simulink model "mbf_3beams"  (R2013a) (continued)
== system mbf_3beams == (continued)
      mask Display:
      | port_label('input', 1, 'd');
      | port_label('output', 1, 'q');
      | disp(['Z^-', num2str(latency)]);
  pipeline32 : Subsystem  AncestorBlock=casper_library_delays/pipeline  mask(pipeline){latency=1}
      mask help:
      | An explicitly laid-out delay line to avoid mapping to SRL16s
      | for use in pipelining to help achieve timing closure.
      mask init:
      | pipeline_init(gcb,'latency',latency);
      settings: AttributesFormatString=Latency = %<latency>
      mask Display:
      | port_label('input', 1, 'd');
      | port_label('output', 1, 'q');
      | disp(['Z^-', num2str(latency)]);
  pipeline33 : Subsystem  AncestorBlock=casper_library_delays/pipeline  mask(pipeline){latency=1}
      mask help:
      | An explicitly laid-out delay line to avoid mapping to SRL16s
      | for use in pipelining to help achieve timing closure.
      mask init:
      | pipeline_init(gcb,'latency',latency);
      settings: AttributesFormatString=Latency = %<latency>
      mask Display:
      | port_label('input', 1, 'd');
      | port_label('output', 1, 'q');
      | disp(['Z^-', num2str(latency)]);
  pipeline34 : Subsystem  AncestorBlock=casper_library_delays/pipeline  mask(pipeline){latency=2}
      mask help:
      | An explicitly laid-out delay line to avoid mapping to SRL16s
      | for use in pipelining to help achieve timing closure.
      mask init:
      | pipeline_init(gcb,'latency',latency);
      settings: AttributesFormatString=Latency = %<latency>
      mask Display:
      | port_label('input', 1, 'd');
      | port_label('output', 1, 'q');
      | disp(['Z^-', num2str(latency)]);
  pipeline35 : casper_library_delays/pipeline  latency=2
      settings: AttributesFormatString=Latency = %<latency>
  pipeline36 : Subsystem  AncestorBlock=casper_library_delays/pipeline  mask(pipeline){latency=2}
      mask help:
      | An explicitly laid-out delay line to avoid mapping to SRL16s
      | for use in pipelining to help achieve timing closure.
      mask init:
      | pipeline_init(gcb,'latency',latency);
      settings: AttributesFormatString=Latency = %<latency>
      mask Display:
      | port_label('input', 1, 'd');
      | port_label('output', 1, 'q');
      | disp(['Z^-', num2str(latency)]);
  pipeline37 : Subsystem  AncestorBlock=casper_library_delays/pipeline  mask(pipeline){latency=2}
      mask help:
      | An explicitly laid-out delay line to avoid mapping to SRL16s
      | for use in pipelining to help achieve timing closure.
      mask init:
      | pipeline_init(gcb,'latency',latency);
      settings: AttributesFormatString=Latency = %<latency>
      mask Display:
      | port_label('input', 1, 'd');
      | port_label('output', 1, 'q');
      | disp(['Z^-', num2str(latency)]);
  pipeline38 : Subsystem  AncestorBlock=casper_library_delays/pipeline  mask(pipeline){latency=2}
      mask help:
      | An explicitly laid-out delay line to avoid mapping to SRL16s
      | for use in pipelining to help achieve timing closure.
      mask init:
      | pipeline_init(gcb,'latency',latency);
      settings: AttributesFormatString=Latency = %<latency>
      mask Display:
      | port_label('input', 1, 'd');
      | port_label('output', 1, 'q');
      | disp(['Z^-', num2str(latency)]);
  pipeline39 : Subsystem  AncestorBlock=casper_library_delays/pipeline  mask(pipeline){latency=1}
      mask help:
      | An explicitly laid-out delay line to avoid mapping to SRL16s
      | for use in pipelining to help achieve timing closure.
      mask init:
      | pipeline_init(gcb,'latency',latency);
      settings: AttributesFormatString=Latency = %<latency>
      mask Display:
      | port_label('input', 1, 'd');
      | port_label('output', 1, 'q');
      | disp(['Z^-', num2str(latency)]);
  pipeline4 : Subsystem  AncestorBlock=casper_library_delays/pipeline  mask(pipeline){latency=1}
      mask help:
      | An explicitly laid-out delay line to avoid mapping to SRL16s
      | for use in pipelining to help achieve timing closure.
      mask init:
      | pipeline_init(gcb,'latency',latency);
      settings: AttributesFormatString=Latency = %<latency>
      mask Display:
      | port_label('input', 1, 'd');
      | port_label('output', 1, 'q');
      | disp(['Z^-', num2str(latency)]);
  pipeline40 : Subsystem  AncestorBlock=casper_library_delays/pipeline  mask(pipeline){latency=1}
      mask help:
      | An explicitly laid-out delay line to avoid mapping to SRL16s
      | for use in pipelining to help achieve timing closure.
      mask init:
      | pipeline_init(gcb,'latency',latency);
      settings: AttributesFormatString=Latency = %<latency>
      mask Display:
      | port_label('input', 1, 'd');
      | port_label('output', 1, 'q');
      | disp(['Z^-', num2str(latency)]);
  pipeline41 : Subsystem  AncestorBlock=casper_library_delays/pipeline  mask(pipeline){latency=1}
      mask help:
      | An explicitly laid-out delay line to avoid mapping to SRL16s
      | for use in pipelining to help achieve timing closure.
      mask init:
      | pipeline_init(gcb,'latency',latency);
      settings: AttributesFormatString=Latency = %<latency>
      mask Display:
      | port_label('input', 1, 'd');
      | port_label('output', 1, 'q');
      | disp(['Z^-', num2str(latency)]);
  pipeline42 : Subsystem  AncestorBlock=casper_library_delays/pipeline  mask(pipeline){latency=1}
      mask help:
      | An explicitly laid-out delay line to avoid mapping to SRL16s
      | for use in pipelining to help achieve timing closure.
      mask init:
      | pipeline_init(gcb,'latency',latency);
      settings: AttributesFormatString=Latency = %<latency>
      mask Display:
      | port_label('input', 1, 'd');
      | port_label('output', 1, 'q');
      | disp(['Z^-', num2str(latency)]);
  pipeline43 : Subsystem  AncestorBlock=casper_library_delays/pipeline  mask(pipeline){latency=1}
      mask help:
      | An explicitly laid-out delay line to avoid mapping to SRL16s
      | for use in pipelining to help achieve timing closure.
      mask init:
      | pipeline_init(gcb,'latency',latency);
      settings: AttributesFormatString=Latency = %<latency>
      mask Display:
      | port_label('input', 1, 'd');
      | port_label('output', 1, 'q');
      | disp(['Z^-', num2str(latency)]);
  pipeline44 : Subsystem  AncestorBlock=casper_library_delays/pipeline  mask(pipeline){latency=1}
      mask help:
      | An explicitly laid-out delay line to avoid mapping to SRL16s
      | for use in pipelining to help achieve timing closure.
      mask init:
      | pipeline_init(gcb,'latency',latency);
      settings: AttributesFormatString=Latency = %<latency>
      mask Display:
      | port_label('input', 1, 'd');
      | port_label('output', 1, 'q');
      | disp(['Z^-', num2str(latency)]);
  pipeline45 : Subsystem  AncestorBlock=casper_library_delays/pipeline  mask(pipeline){latency=1}
      mask help:
      | An explicitly laid-out delay line to avoid mapping to SRL16s
      | for use in pipelining to help achieve timing closure.
      mask init:
      | pipeline_init(gcb,'latency',latency);
      settings: AttributesFormatString=Latency = %<latency>
      mask Display:
      | port_label('input', 1, 'd');
      | port_label('output', 1, 'q');
      | disp(['Z^-', num2str(latency)]);
  pipeline46 : Subsystem  AncestorBlock=casper_library_delays/pipeline  mask(pipeline){latency=1}
      mask help:
      | An explicitly laid-out delay line to avoid mapping to SRL16s
      | for use in pipelining to help achieve timing closure.
      mask init:
      | pipeline_init(gcb,'latency',latency);
      settings: AttributesFormatString=Latency = %<latency>
      mask Display:
      | port_label('input', 1, 'd');
      | port_label('output', 1, 'q');
      | disp(['Z^-', num2str(latency)]);
  pipeline47 : Subsystem  AncestorBlock=casper_library_delays/pipeline  mask(pipeline){latency=1}
      mask help:
      | An explicitly laid-out delay line to avoid mapping to SRL16s
      | for use in pipelining to help achieve timing closure.
      mask init:
      | pipeline_init(gcb,'latency',latency);
      settings: AttributesFormatString=Latency = %<latency>
      mask Display:
      | port_label('input', 1, 'd');
      | port_label('output', 1, 'q');
      | disp(['Z^-', num2str(latency)]);
  pipeline48 : Subsystem  AncestorBlock=casper_library_delays/pipeline  mask(pipeline){latency=1}
      mask help:
      | An explicitly laid-out delay line to avoid mapping to SRL16s
      | for use in pipelining to help achieve timing closure.
      mask init:
      | pipeline_init(gcb,'latency',latency);
      settings: AttributesFormatString=Latency = %<latency>
      mask Display:
      | port_label('input', 1, 'd');
      | port_label('output', 1, 'q');
      | disp(['Z^-', num2str(latency)]);
  pipeline49 : Subsystem  AncestorBlock=casper_library_delays/pipeline  mask(pipeline){latency=1}
      mask help:
      | An explicitly laid-out delay line to avoid mapping to SRL16s
      | for use in pipelining to help achieve timing closure.
      mask init:
      | pipeline_init(gcb,'latency',latency);
      settings: AttributesFormatString=Latency = %<latency>
      mask Display:
      | port_label('input', 1, 'd');
      | port_label('output', 1, 'q');
      | disp(['Z^-', num2str(latency)]);
  pipeline5 : Subsystem  AncestorBlock=casper_library_delays/pipeline  mask(pipeline){latency=1}
      mask help:
      | An explicitly laid-out delay line to avoid mapping to SRL16s
      | for use in pipelining to help achieve timing closure.
      mask init:
      | pipeline_init(gcb,'latency',latency);
      settings: AttributesFormatString=Latency = %<latency>
      mask Display:
      | port_label('input', 1, 'd');
      | port_label('output', 1, 'q');
      | disp(['Z^-', num2str(latency)]);
  pipeline50 : Subsystem  AncestorBlock=casper_library_delays/pipeline  mask(pipeline){latency=1}
      mask help:
      | An explicitly laid-out delay line to avoid mapping to SRL16s
      | for use in pipelining to help achieve timing closure.
      mask init:
      | pipeline_init(gcb,'latency',latency);
      settings: AttributesFormatString=Latency = %<latency>
      mask Display:
      | port_label('input', 1, 'd');
      | port_label('output', 1, 'q');
      | disp(['Z^-', num2str(latency)]);
  pipeline51 : Subsystem  AncestorBlock=casper_library_delays/pipeline  mask(pipeline){latency=1}
      mask help:
      | An explicitly laid-out delay line to avoid mapping to SRL16s
      | for use in pipelining to help achieve timing closure.
      mask init:
      | pipeline_init(gcb,'latency',latency);
      settings: AttributesFormatString=Latency = %<latency>
      mask Display:
      | port_label('input', 1, 'd');
      | port_label('output', 1, 'q');
      | disp(['Z^-', num2str(latency)]);
  pipeline52 : Subsystem  AncestorBlock=casper_library_delays/pipeline  mask(pipeline){latency=1}
      mask help:
      | An explicitly laid-out delay line to avoid mapping to SRL16s
      | for use in pipelining to help achieve timing closure.
      mask init:
      | pipeline_init(gcb,'latency',latency);
      settings: AttributesFormatString=Latency = %<latency>
      mask Display:
      | port_label('input', 1, 'd');
      | port_label('output', 1, 'q');
      | disp(['Z^-', num2str(latency)]);
  pipeline53 : Subsystem  AncestorBlock=casper_library_delays/pipeline  mask(pipeline){latency=1}
      mask help:
      | An explicitly laid-out delay line to avoid mapping to SRL16s
      | for use in pipelining to help achieve timing closure.
      mask init:
      | pipeline_init(gcb,'latency',latency);
      settings: AttributesFormatString=Latency = %<latency>
      mask Display:
      | port_label('input', 1, 'd');
      | port_label('output', 1, 'q');
      | disp(['Z^-', num2str(latency)]);
  pipeline54 : Subsystem  AncestorBlock=casper_library_delays/pipeline  mask(pipeline){latency=1}
      mask help:
      | An explicitly laid-out delay line to avoid mapping to SRL16s
      | for use in pipelining to help achieve timing closure.
      mask init:
      | pipeline_init(gcb,'latency',latency);
      settings: AttributesFormatString=Latency = %<latency>
      mask Display:
      | port_label('input', 1, 'd');
      | port_label('output', 1, 'q');
      | disp(['Z^-', num2str(latency)]);
  pipeline55 : Subsystem  AncestorBlock=casper_library_delays/pipeline  mask(pipeline){latency=1}
      mask help:
      | An explicitly laid-out delay line to avoid mapping to SRL16s
      | for use in pipelining to help achieve timing closure.
      mask init:
      | pipeline_init(gcb,'latency',latency);
      settings: AttributesFormatString=Latency = %<latency>
      mask Display:
      | port_label('input', 1, 'd');
      | port_label('output', 1, 'q');
      | disp(['Z^-', num2str(latency)]);
  pipeline56 : Subsystem  AncestorBlock=casper_library_delays/pipeline  mask(pipeline){latency=1}
      mask help:
      | An explicitly laid-out delay line to avoid mapping to SRL16s
      | for use in pipelining to help achieve timing closure.
      mask init:
      | pipeline_init(gcb,'latency',latency);
      settings: AttributesFormatString=Latency = %<latency>
      mask Display:
      | port_label('input', 1, 'd');
      | port_label('output', 1, 'q');
      | disp(['Z^-', num2str(latency)]);
  pipeline57 : Subsystem  AncestorBlock=casper_library_delays/pipeline  mask(pipeline){latency=1}
      mask help:
      | An explicitly laid-out delay line to avoid mapping to SRL16s
      | for use in pipelining to help achieve timing closure.
      mask init:
      | pipeline_init(gcb,'latency',latency);
      settings: AttributesFormatString=Latency = %<latency>
      mask Display:
      | port_label('input', 1, 'd');
      | port_label('output', 1, 'q');
      | disp(['Z^-', num2str(latency)]);
  pipeline58 : Subsystem  AncestorBlock=casper_library_delays/pipeline  mask(pipeline){latency=1}
      mask help:
      | An explicitly laid-out delay line to avoid mapping to SRL16s
      | for use in pipelining to help achieve timing closure.
      mask init:
      | pipeline_init(gcb,'latency',latency);
      settings: AttributesFormatString=Latency = %<latency>
      mask Display:
      | port_label('input', 1, 'd');
      | port_label('output', 1, 'q');
      | disp(['Z^-', num2str(latency)]);
  pipeline59 : Subsystem  AncestorBlock=casper_library_delays/pipeline  mask(pipeline){latency=1}
      mask help:
      | An explicitly laid-out delay line to avoid mapping to SRL16s
      | for use in pipelining to help achieve timing closure.
      mask init:
      | pipeline_init(gcb,'latency',latency);
      settings: AttributesFormatString=Latency = %<latency>
      mask Display:
      | port_label('input', 1, 'd');
      | port_label('output', 1, 'q');
      | disp(['Z^-', num2str(latency)]);
  pipeline6 : Subsystem  AncestorBlock=casper_library_delays/pipeline  mask(pipeline){latency=1}
      mask help:
      | An explicitly laid-out delay line to avoid mapping to SRL16s
      | for use in pipelining to help achieve timing closure.
      mask init:
      | pipeline_init(gcb,'latency',latency);
      settings: AttributesFormatString=Latency = %<latency>
      mask Display:
      | port_label('input', 1, 'd');
      | port_label('output', 1, 'q');
      | disp(['Z^-', num2str(latency)]);
  pipeline60 : Subsystem  AncestorBlock=casper_library_delays/pipeline  mask(pipeline){latency=1}
      mask help:
      | An explicitly laid-out delay line to avoid mapping to SRL16s
      | for use in pipelining to help achieve timing closure.
      mask init:
      | pipeline_init(gcb,'latency',latency);
      settings: AttributesFormatString=Latency = %<latency>
      mask Display:
      | port_label('input', 1, 'd');
      | port_label('output', 1, 'q');
      | disp(['Z^-', num2str(latency)]);
  pipeline61 : Subsystem  AncestorBlock=casper_library_delays/pipeline  mask(pipeline){latency=1}
      mask help:
      | An explicitly laid-out delay line to avoid mapping to SRL16s
      | for use in pipelining to help achieve timing closure.
      mask init:
      | pipeline_init(gcb,'latency',latency);
      settings: AttributesFormatString=Latency = %<latency>
      mask Display:
      | port_label('input', 1, 'd');
      | port_label('output', 1, 'q');
      | disp(['Z^-', num2str(latency)]);
  pipeline62 : Subsystem  AncestorBlock=casper_library_delays/pipeline  mask(pipeline){latency=1}
      mask help:
      | An explicitly laid-out delay line to avoid mapping to SRL16s
      | for use in pipelining to help achieve timing closure.
      mask init:
      | pipeline_init(gcb,'latency',latency);
      settings: AttributesFormatString=Latency = %<latency>
      mask Display:
      | port_label('input', 1, 'd');
      | port_label('output', 1, 'q');
      | disp(['Z^-', num2str(latency)]);
  pipeline63 : Subsystem  AncestorBlock=casper_library_delays/pipeline  mask(pipeline){latency=1}
      mask help:
      | An explicitly laid-out delay line to avoid mapping to SRL16s
      | for use in pipelining to help achieve timing closure.
      mask init:
      | pipeline_init(gcb,'latency',latency);
      settings: AttributesFormatString=Latency = %<latency>
      mask Display:
      | port_label('input', 1, 'd');
      | port_label('output', 1, 'q');
      | disp(['Z^-', num2str(latency)]);
  pipeline64 : Subsystem  AncestorBlock=casper_library_delays/pipeline  mask(pipeline){latency=1}
      mask help:
      | An explicitly laid-out delay line to avoid mapping to SRL16s
      | for use in pipelining to help achieve timing closure.
      mask init:
      | pipeline_init(gcb,'latency',latency);
      settings: AttributesFormatString=Latency = %<latency>
      mask Display:
      | port_label('input', 1, 'd');
      | port_label('output', 1, 'q');
      | disp(['Z^-', num2str(latency)]);
  pipeline65 : Subsystem  AncestorBlock=casper_library_delays/pipeline  mask(pipeline){latency=1}
      mask help:
      | An explicitly laid-out delay line to avoid mapping to SRL16s
      | for use in pipelining to help achieve timing closure.
      mask init:
      | pipeline_init(gcb,'latency',latency);
      settings: AttributesFormatString=Latency = %<latency>
      mask Display:
      | port_label('input', 1, 'd');
      | port_label('output', 1, 'q');
      | disp(['Z^-', num2str(latency)]);
  pipeline66 : Subsystem  AncestorBlock=casper_library_delays/pipeline  mask(pipeline){latency=1}
      mask help:
      | An explicitly laid-out delay line to avoid mapping to SRL16s
      | for use in pipelining to help achieve timing closure.
      mask init:
      | pipeline_init(gcb,'latency',latency);
      settings: AttributesFormatString=Latency = %<latency>
      mask Display:
      | port_label('input', 1, 'd');
      | port_label('output', 1, 'q');
      | disp(['Z^-', num2str(latency)]);
  pipeline67 : Subsystem  AncestorBlock=casper_library_delays/pipeline  mask(pipeline){latency=1}
      mask help:
      | An explicitly laid-out delay line to avoid mapping to SRL16s
      | for use in pipelining to help achieve timing closure.
      mask init:
      | pipeline_init(gcb,'latency',latency);
      settings: AttributesFormatString=Latency = %<latency>
      mask Display:
      | port_label('input', 1, 'd');
      | port_label('output', 1, 'q');
      | disp(['Z^-', num2str(latency)]);
  pipeline68 : Subsystem  AncestorBlock=casper_library_delays/pipeline  mask(pipeline){latency=1}
      mask help:
      | An explicitly laid-out delay line to avoid mapping to SRL16s
      | for use in pipelining to help achieve timing closure.
      mask init:
      | pipeline_init(gcb,'latency',latency);
      settings: AttributesFormatString=Latency = %<latency>
      mask Display:
      | port_label('input', 1, 'd');
      | port_label('output', 1, 'q');
      | disp(['Z^-', num2str(latency)]);
  pipeline69 : Subsystem  AncestorBlock=casper_library_delays/pipeline  mask(pipeline){latency=1}
      mask help:
      | An explicitly laid-out delay line to avoid mapping to SRL16s
      | for use in pipelining to help achieve timing closure.
      mask init:
      | pipeline_init(gcb,'latency',latency);
      settings: AttributesFormatString=Latency = %<latency>
      mask Display:
      | port_label('input', 1, 'd');
      | port_label('output', 1, 'q');
      | disp(['Z^-', num2str(latency)]);
  pipeline7 : Subsystem  AncestorBlock=casper_library_delays/pipeline  mask(pipeline){latency=1}
      mask help:
      | An explicitly laid-out delay line to avoid mapping to SRL16s
      | for use in pipelining to help achieve timing closure.
      mask init:
      | pipeline_init(gcb,'latency',latency);
      settings: AttributesFormatString=Latency = %<latency>
      mask Display:
      | port_label('input', 1, 'd');
      | port_label('output', 1, 'q');
      | disp(['Z^-', num2str(latency)]);
  pipeline70 : Subsystem  AncestorBlock=casper_library_delays/pipeline  mask(pipeline){latency=1}
      mask help:
      | An explicitly laid-out delay line to avoid mapping to SRL16s
      | for use in pipelining to help achieve timing closure.
      mask init:
      | pipeline_init(gcb,'latency',latency);
      settings: AttributesFormatString=Latency = %<latency>
      mask Display:
      | port_label('input', 1, 'd');
      | port_label('output', 1, 'q');
      | disp(['Z^-', num2str(latency)]);
  pipeline71 : Subsystem  AncestorBlock=casper_library_delays/pipeline  mask(pipeline){latency=1}
      mask help:
      | An explicitly laid-out delay line to avoid mapping to SRL16s
      | for use in pipelining to help achieve timing closure.
      mask init:
      | pipeline_init(gcb,'latency',latency);
      settings: AttributesFormatString=Latency = %<latency>
      mask Display:
      | port_label('input', 1, 'd');
      | port_label('output', 1, 'q');
      | disp(['Z^-', num2str(latency)]);
  pipeline72 : Subsystem  AncestorBlock=casper_library_delays/pipeline  mask(pipeline){latency=1}
      mask help:
      | An explicitly laid-out delay line to avoid mapping to SRL16s
      | for use in pipelining to help achieve timing closure.
      mask init:
      | pipeline_init(gcb,'latency',latency);
      settings: AttributesFormatString=Latency = %<latency>
      mask Display:
      | port_label('input', 1, 'd');
      | port_label('output', 1, 'q');
      | disp(['Z^-', num2str(latency)]);
  pipeline73 : Subsystem  AncestorBlock=casper_library_delays/pipeline  mask(pipeline){latency=1}
      mask help:
      | An explicitly laid-out delay line to avoid mapping to SRL16s
      | for use in pipelining to help achieve timing closure.
      mask init:
      | pipeline_init(gcb,'latency',latency);
      settings: AttributesFormatString=Latency = %<latency>
      mask Display:
      | port_label('input', 1, 'd');
      | port_label('output', 1, 'q');
      | disp(['Z^-', num2str(latency)]);
  pipeline74 : Subsystem  AncestorBlock=casper_library_delays/pipeline  mask(pipeline){latency=1}
      mask help:
      | An explicitly laid-out delay line to avoid mapping to SRL16s
      | for use in pipelining to help achieve timing closure.
      mask init:
      | pipeline_init(gcb,'latency',latency);
      settings: AttributesFormatString=Latency = %<latency>
      mask Display:
      | port_label('input', 1, 'd');
      | port_label('output', 1, 'q');
      | disp(['Z^-', num2str(latency)]);
  pipeline75 : Subsystem  AncestorBlock=casper_library_delays/pipeline  mask(pipeline){latency=1}
      mask help:
      | An explicitly laid-out delay line to avoid mapping to SRL16s
      | for use in pipelining to help achieve timing closure.
      mask init:
      | pipeline_init(gcb,'latency',latency);
      settings: AttributesFormatString=Latency = %<latency>
      mask Display:
      | port_label('input', 1, 'd');
      | port_label('output', 1, 'q');
      | disp(['Z^-', num2str(latency)]);
  pipeline76 : Subsystem  AncestorBlock=casper_library_delays/pipeline  mask(pipeline){latency=1}
      mask help:
      | An explicitly laid-out delay line to avoid mapping to SRL16s
      | for use in pipelining to help achieve timing closure.
      mask init:
      | pipeline_init(gcb,'latency',latency);
      settings: AttributesFormatString=Latency = %<latency>
      mask Display:
      | port_label('input', 1, 'd');
      | port_label('output', 1, 'q');
      | disp(['Z^-', num2str(latency)]);
  pipeline77 : Subsystem  AncestorBlock=casper_library_delays/pipeline  mask(pipeline){latency=1}
      mask help:
      | An explicitly laid-out delay line to avoid mapping to SRL16s
      | for use in pipelining to help achieve timing closure.
      mask init:
      | pipeline_init(gcb,'latency',latency);
      settings: AttributesFormatString=Latency = %<latency>
      mask Display:
      | port_label('input', 1, 'd');
      | port_label('output', 1, 'q');
      | disp(['Z^-', num2str(latency)]);
  pipeline78 : Subsystem  AncestorBlock=casper_library_delays/pipeline  mask(pipeline){latency=1}
      mask help:
      | An explicitly laid-out delay line to avoid mapping to SRL16s
      | for use in pipelining to help achieve timing closure.
      mask init:
      | pipeline_init(gcb,'latency',latency);
      settings: AttributesFormatString=Latency = %<latency>
      mask Display:
      | port_label('input', 1, 'd');
      | port_label('output', 1, 'q');
      | disp(['Z^-', num2str(latency)]);
  pipeline79 : Subsystem  AncestorBlock=casper_library_delays/pipeline  mask(pipeline){latency=1}
      mask help:
      | An explicitly laid-out delay line to avoid mapping to SRL16s
      | for use in pipelining to help achieve timing closure.
      mask init:
      | pipeline_init(gcb,'latency',latency);
      settings: AttributesFormatString=Latency = %<latency>
      mask Display:
      | port_label('input', 1, 'd');
      | port_label('output', 1, 'q');
      | disp(['Z^-', num2str(latency)]);
  pipeline8 : Subsystem  AncestorBlock=casper_library_delays/pipeline  mask(pipeline){latency=1}
      mask help:
      | An explicitly laid-out delay line to avoid mapping to SRL16s
      | for use in pipelining to help achieve timing closure.
      mask init:
      | pipeline_init(gcb,'latency',latency);
      settings: AttributesFormatString=Latency = %<latency>
      mask Display:
      | port_label('input', 1, 'd');
      | port_label('output', 1, 'q');
      | disp(['Z^-', num2str(latency)]);
  pipeline80 : Subsystem  AncestorBlock=casper_library_delays/pipeline  mask(pipeline){latency=1}
      mask help:
      | An explicitly laid-out delay line to avoid mapping to SRL16s
      | for use in pipelining to help achieve timing closure.
      mask init:
      | pipeline_init(gcb,'latency',latency);
      settings: AttributesFormatString=Latency = %<latency>
      mask Display:
      | port_label('input', 1, 'd');
      | port_label('output', 1, 'q');
      | disp(['Z^-', num2str(latency)]);
  pipeline81 : Subsystem  AncestorBlock=casper_library_delays/pipeline  mask(pipeline){latency=1}
      mask help:
      | An explicitly laid-out delay line to avoid mapping to SRL16s
      | for use in pipelining to help achieve timing closure.
      mask init:
      | pipeline_init(gcb,'latency',latency);
      settings: AttributesFormatString=Latency = %<latency>
      mask Display:
      | port_label('input', 1, 'd');
      | port_label('output', 1, 'q');
      | disp(['Z^-', num2str(latency)]);
  pipeline82 : Subsystem  AncestorBlock=casper_library_delays/pipeline  mask(pipeline){latency=1}
      mask help:
      | An explicitly laid-out delay line to avoid mapping to SRL16s
      | for use in pipelining to help achieve timing closure.
      mask init:
      | pipeline_init(gcb,'latency',latency);
      settings: AttributesFormatString=Latency = %<latency>
      mask Display:
      | port_label('input', 1, 'd');
      | port_label('output', 1, 'q');
      | disp(['Z^-', num2str(latency)]);
  pipeline83 : Subsystem  AncestorBlock=casper_library_delays/pipeline  mask(pipeline){latency=1}
      mask help:
      | An explicitly laid-out delay line to avoid mapping to SRL16s
      | for use in pipelining to help achieve timing closure.
      mask init:
      | pipeline_init(gcb,'latency',latency);
      settings: AttributesFormatString=Latency = %<latency>
      mask Display:
      | port_label('input', 1, 'd');
      | port_label('output', 1, 'q');
      | disp(['Z^-', num2str(latency)]);
  pipeline84 : Subsystem  AncestorBlock=casper_library_delays/pipeline  mask(pipeline){latency=1}
      mask help:
      | An explicitly laid-out delay line to avoid mapping to SRL16s
      | for use in pipelining to help achieve timing closure.
      mask init:
      | pipeline_init(gcb,'latency',latency);
      settings: AttributesFormatString=Latency = %<latency>
      mask Display:
      | port_label('input', 1, 'd');
      | port_label('output', 1, 'q');
      | disp(['Z^-', num2str(latency)]);
  pipeline85 : Subsystem  AncestorBlock=casper_library_delays/pipeline  mask(pipeline){latency=1}
      mask help:
      | An explicitly laid-out delay line to avoid mapping to SRL16s
      | for use in pipelining to help achieve timing closure.
      mask init:
      | pipeline_init(gcb,'latency',latency);
      settings: AttributesFormatString=Latency = %<latency>
      mask Display:
      | port_label('input', 1, 'd');
      | port_label('output', 1, 'q');
      | disp(['Z^-', num2str(latency)]);
  pipeline86 : Subsystem  AncestorBlock=casper_library_delays/pipeline  mask(pipeline){latency=1}
      mask help:
      | An explicitly laid-out delay line to avoid mapping to SRL16s
      | for use in pipelining to help achieve timing closure.
      mask init:
      | pipeline_init(gcb,'latency',latency);
      settings: AttributesFormatString=Latency = %<latency>
      mask Display:
      | port_label('input', 1, 'd');
      | port_label('output', 1, 'q');
      | disp(['Z^-', num2str(latency)]);
  pipeline87 : Subsystem  AncestorBlock=casper_library_delays/pipeline  mask(pipeline){latency=1}
      mask help:
      | An explicitly laid-out delay line to avoid mapping to SRL16s
      | for use in pipelining to help achieve timing closure.
      mask init:
      | pipeline_init(gcb,'latency',latency);
      settings: AttributesFormatString=Latency = %<latency>
      mask Display:
      | port_label('input', 1, 'd');
      | port_label('output', 1, 'q');
      | disp(['Z^-', num2str(latency)]);
  pipeline88 : Subsystem  AncestorBlock=casper_library_delays/pipeline  mask(pipeline){latency=1}
      mask help:
      | An explicitly laid-out delay line to avoid mapping to SRL16s
      | for use in pipelining to help achieve timing closure.
      mask init:
      | pipeline_init(gcb,'latency',latency);
      settings: AttributesFormatString=Latency = %<latency>
      mask Display:
      | port_label('input', 1, 'd');
      | port_label('output', 1, 'q');
      | disp(['Z^-', num2str(latency)]);
  pipeline89 : Subsystem  AncestorBlock=casper_library_delays/pipeline  mask(pipeline){latency=1}
      mask help:
      | An explicitly laid-out delay line to avoid mapping to SRL16s
      | for use in pipelining to help achieve timing closure.
      mask init:
      | pipeline_init(gcb,'latency',latency);
      settings: AttributesFormatString=Latency = %<latency>
      mask Display:
      | port_label('input', 1, 'd');
      | port_label('output', 1, 'q');
      | disp(['Z^-', num2str(latency)]);
  pipeline9 : Subsystem  AncestorBlock=casper_library_delays/pipeline  mask(pipeline){latency=1}
      mask help:
      | An explicitly laid-out delay line to avoid mapping to SRL16s
      | for use in pipelining to help achieve timing closure.
      mask init:
      | pipeline_init(gcb,'latency',latency);
      settings: AttributesFormatString=Latency = %<latency>
      mask Display:
      | port_label('input', 1, 'd');
      | port_label('output', 1, 'q');
      | disp(['Z^-', num2str(latency)]);
  pipeline90 : Subsystem  AncestorBlock=casper_library_delays/pipeline  mask(pipeline){latency=1}
      mask help:
      | An explicitly laid-out delay line to avoid mapping to SRL16s
      | for use in pipelining to help achieve timing closure.
      mask init:
      | pipeline_init(gcb,'latency',latency);
      settings: AttributesFormatString=Latency = %<latency>
      mask Display:
      | port_label('input', 1, 'd');
      | port_label('output', 1, 'q');
      | disp(['Z^-', num2str(latency)]);
  pipeline91 : Subsystem  AncestorBlock=casper_library_delays/pipeline  mask(pipeline){latency=1}
      mask help:
      | An explicitly laid-out delay line to avoid mapping to SRL16s
      | for use in pipelining to help achieve timing closure.
      mask init:
      | pipeline_init(gcb,'latency',latency);
      settings: AttributesFormatString=Latency = %<latency>
      mask Display:
      | port_label('input', 1, 'd');
      | port_label('output', 1, 'q');
      | disp(['Z^-', num2str(latency)]);
  pipeline92 : Subsystem  AncestorBlock=casper_library_delays/pipeline  mask(pipeline){latency=1}
      mask help:
      | An explicitly laid-out delay line to avoid mapping to SRL16s
      | for use in pipelining to help achieve timing closure.
      mask init:
      | pipeline_init(gcb,'latency',latency);
      settings: AttributesFormatString=Latency = %<latency>
      mask Display:
      | port_label('input', 1, 'd');
      | port_label('output', 1, 'q');
      | disp(['Z^-', num2str(latency)]);
  pipeline93 : Subsystem  AncestorBlock=casper_library_delays/pipeline  mask(pipeline){latency=1}
      mask help:
      | An explicitly laid-out delay line to avoid mapping to SRL16s
      | for use in pipelining to help achieve timing closure.
      mask init:
      | pipeline_init(gcb,'latency',latency);
      settings: AttributesFormatString=Latency = %<latency>
      mask Display:
      | port_label('input', 1, 'd');
      | port_label('output', 1, 'q');
      | disp(['Z^-', num2str(latency)]);
  pipeline94 : Subsystem  AncestorBlock=casper_library_delays/pipeline  mask(pipeline){latency=1}
      mask help:
      | An explicitly laid-out delay line to avoid mapping to SRL16s
      | for use in pipelining to help achieve timing closure.
      mask init:
      | pipeline_init(gcb,'latency',latency);
      settings: AttributesFormatString=Latency = %<latency>
      mask Display:
      | port_label('input', 1, 'd');
      | port_label('output', 1, 'q');
      | disp(['Z^-', num2str(latency)]);
  pipeline95 : Subsystem  AncestorBlock=casper_library_delays/pipeline  mask(pipeline){latency=1}
      mask help:
      | An explicitly laid-out delay line to avoid mapping to SRL16s
      | for use in pipelining to help achieve timing closure.
      mask init:
      | pipeline_init(gcb,'latency',latency);
      settings: AttributesFormatString=Latency = %<latency>
      mask Display:
      | port_label('input', 1, 'd');
      | port_label('output', 1, 'q');
      | disp(['Z^-', num2str(latency)]);
  pipeline96 : Subsystem  AncestorBlock=casper_library_delays/pipeline  mask(pipeline){latency=1}
      mask help:
      | An explicitly laid-out delay line to avoid mapping to SRL16s
      | for use in pipelining to help achieve timing closure.
      mask init:
      | pipeline_init(gcb,'latency',latency);
      settings: AttributesFormatString=Latency = %<latency>
      mask Display:
      | port_label('input', 1, 'd');
      | port_label('output', 1, 'q');
      | disp(['Z^-', num2str(latency)]);
  pipeline97 : Subsystem  AncestorBlock=casper_library_delays/pipeline  mask(pipeline){latency=1}
      mask help:
      | An explicitly laid-out delay line to avoid mapping to SRL16s
      | for use in pipelining to help achieve timing closure.
      mask init:
      | pipeline_init(gcb,'latency',latency);
      settings: AttributesFormatString=Latency = %<latency>
      mask Display:
      | port_label('input', 1, 'd');
      | port_label('output', 1, 'q');
      | disp(['Z^-', num2str(latency)]);
  pipeline98 : Subsystem  AncestorBlock=casper_library_delays/pipeline  mask(pipeline){latency=1}
      mask help:
      | An explicitly laid-out delay line to avoid mapping to SRL16s
      | for use in pipelining to help achieve timing closure.
      mask init:
      | pipeline_init(gcb,'latency',latency);
      settings: AttributesFormatString=Latency = %<latency>
      mask Display:
      | port_label('input', 1, 'd');
      | port_label('output', 1, 'q');
      | disp(['Z^-', num2str(latency)]);
  pipeline99 : Subsystem  AncestorBlock=casper_library_delays/pipeline  mask(pipeline){latency=1}
      mask help:
      | An explicitly laid-out delay line to avoid mapping to SRL16s
      | for use in pipelining to help achieve timing closure.
      mask init:
      | pipeline_init(gcb,'latency',latency);
      settings: AttributesFormatString=Latency = %<latency>
      mask Display:
      | port_label('input', 1, 'd');
      | port_label('output', 1, 'q');
      | disp(['Z^-', num2str(latency)]);
  power_adc : Subsystem
  ri_to_c : casper_library_misc/ri_to_c
  ri_to_c1 : casper_library_misc/ri_to_c
  snap_adc : Subsystem
  sync_gen : Subsystem  AncestorBlock=casper_library_misc/sync_gen  mask(sync_gen){sim_acc_len=1, fft_size=256, fft_simult_inputs=1, pfb_fir_taps=4, reorder_vec=[2 2 2], scale=1}
      mask prompts: sim_acc_len "Simulation Accumulation Length"; fft_size "FFT Size"; fft_simult_inputs "Simultaneous Inputs (FFT)"; pfb_fir_taps "Taps in pfb_fir (use 1 if no pfb_fir)"; reorder_vec "Reorder Orders (e.g. [2 2 2])"
      mask help:
      | This block computes the sync period based on system parameters as
      | described in Casper memo.  Detailed descritions of the block's
      | variables can be found in the 'Block Documentation' section of
      | the CASPER wiki.
      mask init:
      | sync_gen_init(gcb, ...
      |     'sim_acc_len', sim_acc_len, ...
      |     'fft_size', fft_size, ...
      |     'fft_simult_inputs', fft_simult_inputs,...
      |     'pfb_fir_taps', pfb_fir_taps, ...
      |     'reorder_vec', reorder_vec, ...
      |     'scale', scale);
      settings: AttributesFormatString=sim_sync_period=2048
      mask Help:
      | SyncPeriod=LCM(reorder_vec)*(acc_num)*(pfb_fir_len)*(fft_size/fft_simult_inputs)
      | 
      | See CASPER sync pulse memo for more details.
  tag scope: hold_data=global
  wires:
  adc16x250-8/3(a3) -> Bus Creator/3
  adc16x250-8/2(a2) -> Bus Creator/2
  adc16x250-8/4(a4) -> Bus Creator/4
  adc16x250-8/1(a1) -> Bus Creator/1
  Bus Creator/1 -> [adc16_chan_a]
  adc16x250-8/8(b4) -> Bus Creator1/4
  adc16x250-8/6(b2) -> Bus Creator1/2
  adc16x250-8/7(b3) -> Bus Creator1/3
  adc16x250-8/5(b1) -> Bus Creator1/1
  adc16x250-8/12(c4) -> Bus Creator2/4
  adc16x250-8/11(c3) -> Bus Creator2/3
  adc16x250-8/10(c2) -> Bus Creator2/2
  adc16x250-8/9(c1) -> Bus Creator2/1
  adc16x250-8/15(d3) -> Bus Creator3/3
  adc16x250-8/16(d4) -> Bus Creator3/4
  adc16x250-8/14(d2) -> Bus Creator3/2
  adc16x250-8/13(d1) -> Bus Creator3/1
  Bus Creator1/1 -> [adc16_chan_b]
  Bus Creator2/1 -> [adc16_chan_c]
  Bus Creator3/1 -> [adc16_chan_d]
  Gain/1 -> adc16x250-8/1(a1_sim)
  Gain1/1 -> adc16x250-8/2(a2_sim)
  Gain2/1 -> adc16x250-8/3(a3_sim)
  Gain3/1 -> adc16x250-8/4(a4_sim)
  Gain4/1 -> adc16x250-8/5(b1_sim)
  Gain5/1 -> adc16x250-8/6(b2_sim)
  Gain6/1 -> adc16x250-8/7(b3_sim)
  Gain7/1 -> adc16x250-8/8(b4_sim)
  Gain10/1 -> adc16x250-8/11(c3_sim)
  Gain8/1 -> adc16x250-8/9(c1_sim)
  Gain12/1 -> adc16x250-8/13(d1_sim)
  Gain15/1 -> adc16x250-8/16(d4_sim)
  Gain11/1 -> adc16x250-8/12(c4_sim)
  Gain14/1 -> adc16x250-8/15(d3_sim)
  Gain9/1 -> adc16x250-8/10(c2_sim)
  Gain13/1 -> adc16x250-8/14(d2_sim)
  [adc16_chan_a] -> Bus Selector/1
  generators/1(Out1) -> Gain/1
  generators/2(Out2) -> Gain1/1
  generators/3(Out3) -> Gain2/1
  generators/4(Out4) -> Gain3/1
  generators/5(Out5) -> Gain4/1
  generators/6(Out6) -> Gain5/1
  generators/7(Out7) -> Gain6/1
  generators/8(Out8) -> Gain7/1
  generators/9(Out9) -> Gain8/1
  generators/10(Out10) -> Gain9/1
  generators/11(Out11) -> Gain10/1
  generators/12(Out12) -> Gain11/1
  generators/13(Out13) -> Gain12/1
  generators/14(Out14) -> Gain13/1
  generators/15(Out15) -> Gain14/1
  generators/16(Out16) -> Gain15/1
  sync_gen/1(sync_out) -> pipeline34/1(d)
  pipeline34/1(q) -> [sync_gen]
  cal_acc_len/1(in_reg) -> pipeline67/1(d)
  cal_gain/1(in_reg) -> pipeline66/1(d)
  cal_cnt_rst/1(in_reg) -> pipeline68/1(d)
  Constant2/1 -> snap_adc/5(trig)
  [adc16_chan_c] -> snap_adc/3(data_c)
  [adc16_chan_b] -> snap_adc/2(data_b)
  [adc16_chan_d] -> snap_adc/4(data_d)
  [adc16_chan_a] -> snap_adc/1(data_a)
  Constant3/1 -> power_adc/5(acc_len)
  [adc16_chan_a] -> power_adc/1(chan_a)
  [adc16_chan_b] -> power_adc/2(chan_b)
  [adc16_chan_c] -> power_adc/3(chan_c)
  [adc16_chan_d] -> power_adc/4(chan_d)
  cal_phase_re/1(in_reg) -> Reinterpret/1
  cal_phase_im/1(in_reg) -> Reinterpret17/1
  Reinterpret/1 -> Convert/1
  Reinterpret17/1 -> Convert1/1
  Convert/1 -> ri_to_c/1
  Convert1/1 -> ri_to_c/2
  ri_to_c/1 -> [cal_phase_val]
  Slice2/1 -> edge_detect4/1
  pipeline35/1 -> Slice2/1
  hold_data/1(in_reg) -> pipeline35/1
  edge_detect4/1 -> [hold_data]
  cal_phase_we/1(in_reg) -> edge_detect2/1
  cal_phase_addr/1(in_reg) -> pipeline/1(d)
  edge_detect2/1 -> pipeline2/1(d)
  pipeline/1(q) -> [cal_phase_addr]
  pipeline2/1(q) -> [cal_phase_we]
  [hold_data] -> power_adc/6(trig)
  bf_addr_x/1(in_reg) -> pipeline36/1(d)
  pipeline36/1(q) -> [bf_addr_x]
  bf_addr_y/1(in_reg) -> pipeline37/1(d)
  pipeline37/1(q) -> [bf_addr_y]
  bf_addr_sub/1(in_reg) -> pipeline38/1(d)
  pipeline38/1(q) -> [bf_addr_sub]
  [cal_phase_val] -> cal_phase_bank/1(phase_val)
  [cal_phase_addr] -> cal_phase_bank/2(addr)
  [cal_phase_we] -> cal_phase_bank/3(we)
  beamformer0/1(sync_out) -> [sync_bf]
  [a4] -> pipeline24/1(d)
  [a1] -> pipeline28/1(d)
  [a2] -> pipeline27/1(d)
  [a3] -> pipeline26/1(d)
  [sync_fft] -> pipeline23/1(d)
  bf_gain/1(in_reg) -> pipeline70/1(d)
  bf_cnt_rst/1(in_reg) -> pipeline72/1(d)
  [sync_fft] -> pipeline3/1(d)
  [a1] -> pipeline33/1(d)
  [a2] -> pipeline32/1(d)
  [a3] -> pipeline31/1(d)
  [a4] -> pipeline30/1(d)
  [cal_gain] -> cal_probe0/7(gain)
  cal_new_acc/1(in_reg) -> pipeline69/1(d)
  [sync_gen] -> fft0/1(sync)
  Bus Selector/1 -> fft0/2(pol0_in)  "<a1>"
  Bus Selector/2 -> fft0/3(pol1_in)  "<a2>"
  Bus Selector/3 -> fft0/4(pol2_in)  "<a3>"
  Bus Selector/4 -> fft0/5(pol3_in)  "<a4>"
  fft0/1(sync_out) -> pipeline44/1(d)
  fft0/2(out0) -> pipeline58/1(d)
  fft0/3(out1) -> pipeline57/1(d)
  fft0/4(out2) -> pipeline46/1(d)
  fft0/5(out3) -> pipeline45/1(d)
  bf_phase_re/1(in_reg) -> Reinterpret1/1
  bf_phase_im/1(in_reg) -> Reinterpret2/1
  Reinterpret1/1 -> Convert2/1
  Reinterpret2/1 -> Convert3/1
  Convert2/1 -> ri_to_c1/1
  Convert3/1 -> ri_to_c1/2
  ri_to_c1/1 -> [bf_phase_val]
  bf_phase_we/1(in_reg) -> edge_detect1/1
  edge_detect1/1 -> pipeline1/1(d)
  pipeline1/1(q) -> [bf_phase_we]
  [cal_acc_len] -> acc_control/2(acc_len)
  [cal_cnt_rst] -> acc_control/3(rst)
  [cal_new_acc] -> acc_control/4(new_acc)
  [probe_valid] -> acc_control/5(valid)
  acc_control/2(ctrl_addr) -> [ctrl_addr]
  acc_control/3(ctrl_we) -> [ctrl_we]
  [ctrl_addr] -> cal_probe0/9(ctrl_addr)
  [ctrl_we] -> cal_probe0/8(ctrl_we)
  [ctrl_new_acc] -> cal_probe0/10(ctrl_new_acc)
  acc_control/1(ctrl_new_acc) -> [ctrl_new_acc]
  cal_probe0/2(valid) -> [probe_valid]
  cal_probe0/1(sync_out) -> [sync_probe]
  [sync_probe] -> acc_control/1(sync)
  cal_phase_bank/1(pa1) -> Bus Creator4/1
  cal_phase_bank/2(pa2) -> Bus Creator4/2
  cal_phase_bank/3(pa3) -> Bus Creator4/3
  cal_phase_bank/4(pa4) -> Bus Creator4/4
  Bus Creator4/1 -> [phase_cal_a]
  Bus Creator5/1 -> [phase_cal_b]
  cal_phase_bank/8(pb4) -> Bus Creator5/4
  cal_phase_bank/6(pb2) -> Bus Creator5/2
  cal_phase_bank/5(pb1) -> Bus Creator5/1
  cal_phase_bank/7(pb3) -> Bus Creator5/3
  cal_phase_bank/10(pc2) -> Bus Creator6/2
  cal_phase_bank/9(pc1) -> Bus Creator6/1
  cal_phase_bank/11(pc3) -> Bus Creator6/3
  cal_phase_bank/12(pc4) -> Bus Creator6/4
  cal_phase_bank/15(pd3) -> Bus Creator7/3
  cal_phase_bank/13(pd1) -> Bus Creator7/1
  cal_phase_bank/16(pd4) -> Bus Creator7/4
  cal_phase_bank/14(pd2) -> Bus Creator7/2
  Bus Creator6/1 -> [phase_cal_c]
  Bus Creator7/1 -> [phase_cal_d]
  [phase_cal_a] -> Bus Selector1/1
  Bus Selector1/1 -> fft0/6(phase0)  "<signal1>"
  Bus Selector1/4 -> fft0/9(phase3)  "<signal4>"
  Bus Selector1/3 -> fft0/8(phase2)  "<signal3>"
  Bus Selector1/2 -> fft0/7(phase1)  "<signal2>"
  [adc16_chan_b] -> Bus Selector2/1
  [sync_gen] -> fft1/1(sync)
  Bus Selector2/1 -> fft1/2(pol0_in)  "<b1>"
  Bus Selector2/2 -> fft1/3(pol1_in)  "<b2>"
  Bus Selector2/3 -> fft1/4(pol2_in)  "<b3>"
  Bus Selector2/4 -> fft1/5(pol3_in)  "<b4>"
  fft1/1(sync_out) -> Terminator11/1
  fft1/2(out0) -> pipeline59/1(d)
  fft1/3(out1) -> pipeline63/1(d)
  fft1/4(out2) -> pipeline62/1(d)
  fft1/5(out3) -> pipeline61/1(d)
  [phase_cal_b] -> Bus Selector3/1
  Bus Selector3/1 -> fft1/6(phase0)  "<signal1>"
  Bus Selector3/4 -> fft1/9(phase3)  "<signal4>"
  Bus Selector3/3 -> fft1/8(phase2)  "<signal3>"
  Bus Selector3/2 -> fft1/7(phase1)  "<signal2>"
  [a1] -> pipeline25/1(d)
  [sync_fft] -> pipeline5/1(d)
  [b1] -> pipeline9/1(d)
  [b2] -> pipeline8/1(d)
  [b3] -> pipeline7/1(d)
  [b4] -> pipeline6/1(d)
  [cal_gain] -> cal_probe1/7(gain)
  [ctrl_addr] -> cal_probe1/9(ctrl_addr)
  [ctrl_we] -> cal_probe1/8(ctrl_we)
  [ctrl_new_acc] -> cal_probe1/10(ctrl_new_acc)
  cal_probe1/2(valid) -> Terminator2/1
  cal_probe1/1(sync_out) -> Terminator1/1
  [a1] -> pipeline4/1(d)
  [adc16_chan_c] -> Bus Selector4/1
  [sync_gen] -> fft2/1(sync)
  Bus Selector4/1 -> fft2/2(pol0_in)  "<c1>"
  Bus Selector4/2 -> fft2/3(pol1_in)  "<c2>"
  Bus Selector4/3 -> fft2/4(pol2_in)  "<c3>"
  Bus Selector4/4 -> fft2/5(pol3_in)  "<c4>"
  fft2/1(sync_out) -> Terminator4/1
  fft2/2(out0) -> pipeline60/1(d)
  fft2/3(out1) -> pipeline74/1(d)
  fft2/4(out2) -> pipeline65/1(d)
  fft2/5(out3) -> pipeline64/1(d)
  [phase_cal_c] -> Bus Selector5/1
  Bus Selector5/1 -> fft2/6(phase0)  "<signal1>"
  Bus Selector5/4 -> fft2/9(phase3)  "<signal4>"
  Bus Selector5/3 -> fft2/8(phase2)  "<signal3>"
  Bus Selector5/2 -> fft2/7(phase1)  "<signal2>"
  [sync_fft] -> pipeline11/1(d)
  [c1] -> pipeline15/1(d)
  [c2] -> pipeline14/1(d)
  [c3] -> pipeline13/1(d)
  [c4] -> pipeline12/1(d)
  [cal_gain] -> cal_probe2/7(gain)
  [ctrl_addr] -> cal_probe2/9(ctrl_addr)
  [ctrl_we] -> cal_probe2/8(ctrl_we)
  [ctrl_new_acc] -> cal_probe2/10(ctrl_new_acc)
  cal_probe2/2(valid) -> Terminator5/1
  cal_probe2/1(sync_out) -> Terminator3/1
  [a1] -> pipeline10/1(d)
  [adc16_chan_d] -> Bus Selector6/1
  [sync_gen] -> fft3/1(sync)
  Bus Selector6/1 -> fft3/2(pol0_in)  "<d1>"
  Bus Selector6/2 -> fft3/3(pol1_in)  "<d2>"
  Bus Selector6/3 -> fft3/4(pol2_in)  "<d3>"
  Bus Selector6/4 -> fft3/5(pol3_in)  "<d4>"
  fft3/1(sync_out) -> Terminator7/1
  fft3/2(out0) -> pipeline75/1(d)
  fft3/3(out1) -> pipeline78/1(d)
  fft3/4(out2) -> pipeline77/1(d)
  fft3/5(out3) -> pipeline76/1(d)
  [phase_cal_d] -> Bus Selector7/1
  Bus Selector7/1 -> fft3/6(phase0)  "<signal1>"
  Bus Selector7/4 -> fft3/9(phase3)  "<signal4>"
  Bus Selector7/3 -> fft3/8(phase2)  "<signal3>"
  Bus Selector7/2 -> fft3/7(phase1)  "<signal2>"
  [sync_fft] -> pipeline17/1(d)
  [d1] -> pipeline21/1(d)
  [d2] -> pipeline20/1(d)
  [d3] -> pipeline19/1(d)
  [d4] -> pipeline18/1(d)
  [cal_gain] -> cal_probe3/7(gain)
  [ctrl_addr] -> cal_probe3/9(ctrl_addr)
  [ctrl_we] -> cal_probe3/8(ctrl_we)
  [ctrl_new_acc] -> cal_probe3/10(ctrl_new_acc)
  cal_probe3/2(valid) -> Terminator8/1
  cal_probe3/1(sync_out) -> Terminator6/1
  [a1] -> pipeline16/1(d)
  [b3] -> pipeline51/1(d)
  [b2] -> pipeline47/1(d)
  [b4] -> pipeline50/1(d)
  [b1] -> pipeline22/1(d)
  [d4] -> pipeline53/1(d)
  [d2] -> pipeline55/1(d)
  [d1] -> pipeline56/1(d)
  [d3] -> pipeline54/1(d)
  [c1] -> pipeline49/1(d)
  [c3] -> pipeline29/1(d)
  [c2] -> pipeline48/1(d)
  [c4] -> pipeline52/1(d)
  pipeline25/1(q) -> cal_probe0/6(s_ref)
  pipeline30/1(q) -> cal_probe0/5(in3)
  pipeline31/1(q) -> cal_probe0/4(in2)
  pipeline32/1(q) -> cal_probe0/3(in1)
  pipeline33/1(q) -> cal_probe0/2(in0)
  pipeline3/1(q) -> cal_probe0/1(sync)
  pipeline5/1(q) -> cal_probe1/1(sync)
  pipeline9/1(q) -> cal_probe1/2(in0)
  pipeline7/1(q) -> cal_probe1/4(in2)
  pipeline6/1(q) -> cal_probe1/5(in3)
  pipeline8/1(q) -> cal_probe1/3(in1)
  pipeline4/1(q) -> cal_probe1/6(s_ref)
  pipeline13/1(q) -> cal_probe2/4(in2)
  pipeline11/1(q) -> cal_probe2/1(sync)
  pipeline12/1(q) -> cal_probe2/5(in3)
  pipeline10/1(q) -> cal_probe2/6(s_ref)
  pipeline14/1(q) -> cal_probe2/3(in1)
  pipeline15/1(q) -> cal_probe2/2(in0)
  pipeline16/1(q) -> cal_probe3/6(s_ref)
  pipeline17/1(q) -> cal_probe3/1(sync)
  pipeline19/1(q) -> cal_probe3/4(in2)
  pipeline20/1(q) -> cal_probe3/3(in1)
  pipeline21/1(q) -> cal_probe3/2(in0)
  pipeline18/1(q) -> cal_probe3/5(in3)
  bf_new_acc/1(in_reg) -> pipeline73/1(d)
  [bf_acc_len] -> acc_control_bf/2(acc_len)
  [bf_cnt_rst] -> acc_control_bf/3(rst)
  [bf_new_acc] -> acc_control_bf/4(new_acc)
  [bf_valid] -> acc_control_bf/5(valid)
  acc_control_bf/2(ctrl_addr) -> [ctrl_addr_bf]
  acc_control_bf/3(ctrl_we) -> [ctrl_we_bf]
  acc_control_bf/1(ctrl_new_acc) -> [ctrl_new_acc_bf]
  [bf_sync_probe] -> acc_control_bf/1(sync)
  bf_acc_len/1(in_reg) -> pipeline71/1(d)
  [sync_bf] -> bf_probe0/1(sync)
  [bf0] -> bf_probe0/2(s0)
  [bf_gain] -> bf_probe0/5(gain)
  [ctrl_we_bf] -> bf_probe0/6(we)
  [ctrl_addr_bf] -> bf_probe0/7(addr)
  [ctrl_new_acc_bf] -> bf_probe0/8(new_acc)
  bf_probe0/1(sync_out) -> [bf_sync_probe]
  bf_probe0/2(bf_valid) -> [bf_valid]
  pipeline66/1(q) -> [cal_gain]
  pipeline67/1(q) -> [cal_acc_len]
  pipeline68/1(q) -> [cal_cnt_rst]
  pipeline69/1(q) -> [cal_new_acc]
  pipeline73/1(q) -> [bf_new_acc]
  pipeline72/1(q) -> [bf_cnt_rst]
  pipeline70/1(q) -> [bf_gain]
  pipeline71/1(q) -> [bf_acc_len]
  pipeline23/1(q) -> beamformer0/1(sync)
  pipeline28/1(q) -> beamformer0/2(in1)
  pipeline27/1(q) -> beamformer0/3(in2)
  pipeline26/1(q) -> beamformer0/4(in3)
  pipeline24/1(q) -> beamformer0/5(in4)
  pipeline22/1(q) -> beamformer0/6(in5)
  pipeline47/1(q) -> beamformer0/7(in6)
  pipeline51/1(q) -> beamformer0/8(in7)
  pipeline50/1(q) -> beamformer0/9(in8)
  pipeline49/1(q) -> beamformer0/10(in9)
  pipeline48/1(q) -> beamformer0/11(in10)
  pipeline29/1(q) -> beamformer0/12(in11)
  pipeline52/1(q) -> beamformer0/13(in12)
  pipeline56/1(q) -> beamformer0/14(in13)
  pipeline55/1(q) -> beamformer0/15(in14)
  pipeline54/1(q) -> beamformer0/16(in15)
  pipeline53/1(q) -> beamformer0/17(in16)
  beamformer0/2(out) -> [bf0]
  [bf1] -> bf_probe0/3(s1)
  pipeline44/1(q) -> [sync_fft]
  pipeline58/1(q) -> [a1]
  pipeline57/1(q) -> [a2]
  pipeline46/1(q) -> [a3]
  pipeline45/1(q) -> [a4]
  pipeline61/1(q) -> [b4]
  pipeline59/1(q) -> [b1]
  pipeline62/1(q) -> [b3]
  pipeline63/1(q) -> [b2]
  pipeline64/1(q) -> [c4]
  pipeline60/1(q) -> [c1]
  pipeline74/1(q) -> [c2]
  pipeline65/1(q) -> [c3]
  pipeline76/1(q) -> [d4]
  pipeline78/1(q) -> [d2]
  pipeline77/1(q) -> [d3]
  pipeline75/1(q) -> [d1]
  beamformer1/1(sync_out) -> Terminator/1
  [a4] -> pipeline81/1(d)
  [a1] -> pipeline84/1(d)
  [a2] -> pipeline83/1(d)
  [a3] -> pipeline82/1(d)
  [sync_fft] -> pipeline80/1(d)
  [b3] -> pipeline95/1(d)
  [b2] -> pipeline91/1(d)
  [b4] -> pipeline94/1(d)
  [b1] -> pipeline79/1(d)
  [d4] -> pipeline97/1(d)
  [d2] -> pipeline99/1(d)
  [d1] -> pipeline100/1(d)
  [d3] -> pipeline98/1(d)
  [c1] -> pipeline93/1(d)
  [c3] -> pipeline85/1(d)
  [c2] -> pipeline92/1(d)
  [c4] -> pipeline96/1(d)
  pipeline80/1(q) -> beamformer1/1(sync)
  pipeline84/1(q) -> beamformer1/2(in1)
  pipeline83/1(q) -> beamformer1/3(in2)
  pipeline82/1(q) -> beamformer1/4(in3)
  pipeline81/1(q) -> beamformer1/5(in4)
  pipeline79/1(q) -> beamformer1/6(in5)
  pipeline91/1(q) -> beamformer1/7(in6)
  pipeline95/1(q) -> beamformer1/8(in7)
  pipeline94/1(q) -> beamformer1/9(in8)
  pipeline93/1(q) -> beamformer1/10(in9)
  pipeline92/1(q) -> beamformer1/11(in10)
  pipeline85/1(q) -> beamformer1/12(in11)
  pipeline96/1(q) -> beamformer1/13(in12)
  pipeline100/1(q) -> beamformer1/14(in13)
  pipeline99/1(q) -> beamformer1/15(in14)
  pipeline98/1(q) -> beamformer1/16(in15)
  pipeline97/1(q) -> beamformer1/17(in16)
  beamformer1/2(out) -> [bf1]
  [bf_phase_val] -> beamformer0/18(phase_val)
  [bf_phase_we] -> beamformer0/22(we)
  [bf_addr_y] -> beamformer0/21(addr_y)
  [bf_addr_x] -> beamformer0/20(addr_x)
  [bf_addr_sub] -> beamformer0/19(addr_sub)
  [bf_addr_x] -> beamformer1/20(addr_x)
  [bf_addr_y] -> beamformer1/21(addr_y)
  [bf_addr_sub] -> beamformer1/19(addr_sub)
  [bf_phase_val] -> beamformer1/18(phase_val)
  [bf_phase_we] -> beamformer1/22(we)
  beamformer2/1(sync_out) -> Terminator9/1
  [a4] -> pipeline42/1(d)
  [a1] -> pipeline87/1(d)
  [a2] -> pipeline86/1(d)
  [a3] -> pipeline43/1(d)
  [sync_fft] -> pipeline41/1(d)
  [b3] -> pipeline103/1(d)
  [b2] -> pipeline89/1(d)
  [b4] -> pipeline102/1(d)
  [b1] -> pipeline40/1(d)
  [d4] -> pipeline105/1(d)
  [d2] -> pipeline107/1(d)
  [d1] -> pipeline39/1(d)
  [d3] -> pipeline106/1(d)
  [c1] -> pipeline101/1(d)
  [c3] -> pipeline88/1(d)
  [c2] -> pipeline90/1(d)
  [c4] -> pipeline104/1(d)
  pipeline41/1(q) -> beamformer2/1(sync)
  pipeline87/1(q) -> beamformer2/2(in1)
  pipeline86/1(q) -> beamformer2/3(in2)
  pipeline43/1(q) -> beamformer2/4(in3)
  pipeline42/1(q) -> beamformer2/5(in4)
  pipeline40/1(q) -> beamformer2/6(in5)
  pipeline89/1(q) -> beamformer2/7(in6)
  pipeline103/1(q) -> beamformer2/8(in7)
  pipeline102/1(q) -> beamformer2/9(in8)
  pipeline101/1(q) -> beamformer2/10(in9)
  pipeline90/1(q) -> beamformer2/11(in10)
  pipeline88/1(q) -> beamformer2/12(in11)
  pipeline104/1(q) -> beamformer2/13(in12)
  pipeline39/1(q) -> beamformer2/14(in13)
  pipeline107/1(q) -> beamformer2/15(in14)
  pipeline106/1(q) -> beamformer2/16(in15)
  pipeline105/1(q) -> beamformer2/17(in16)
  beamformer2/2(out) -> [bf2]
  [bf_addr_x] -> beamformer2/20(addr_x)
  [bf_addr_y] -> beamformer2/21(addr_y)
  [bf_addr_sub] -> beamformer2/19(addr_sub)
  [bf_phase_val] -> beamformer2/18(phase_val)
  [bf_phase_we] -> beamformer2/22(we)
  [bf2] -> bf_probe0/4(s2)
